simulink model "sys"  (R2024b)
settings: Solver=ode45  StartTime=0.0  StopTime=3  RelTol=1e-3
values from neighbouring files (symbols this model uses):
  N = Nm+mp*(x2+L*(theta2*cos(theta)-theta1^2*sin(theta)))    [state_equation.m]
  X = nmpc.variable(6,step)    [MPC_controller.m]    (also in MPC_controller_14D.m = nmpc.variable(12, step); mlp.m = Xy(:,1:6)')
  Y = Xy(:,7:8)'    [mlp.m]
undefined symbols (not defined in the file or in its folder): EKF, MPC_controller, Z
libraries: sm_lib x1025, nesl_utility x197, slpidlib x40, simulink x12, dspfdesign x2
(part 26 of 26)

== matlab function sys/Subsystem9/MATLAB Function2 ==
  data: q1 input; theta output; q2 input; phi input
function theta = fcn(q1, q2, phi)

alp = (q2-q1)/2;
theta = alp + q1 -phi;

== matlab function sys/MATLAB Function36 ==
  data: L input; K output
function K = LQR_K(L)

    K = LQR_K(L);

== matlab function sys/MATLAB Function37 ==
  data: State input; T output; K input
function T = Controller(State,K)

    T = Controller(State,K);

== matlab function sys/Subsystem14/MATLAB Function ==
  data: q1 input; L output; q2 input
function L = fcn(q1, q2)
l1 = 0.105;
l2 = 0.125;
alp = (q2-q1)/2;
beta = asin(l1/l2*sin(alp));
theta = pi-alp-beta;
L = l2*sin(theta)/sin(alp);
% L = l1*cos(alp) + l2*cos(beta);

== matlab function sys/Subsystem14/MATLAB Function1 ==
  data: q1 input; L output; q2 input
function L = fcn(q1, q2)
l1 = 0.105;
l2 = 0.125;
alp = (q2-q1)/2;
beta = asin(l1/l2*sin(alp));
theta = pi-alp-beta;
L = l2*sin(theta)/sin(alp);

== matlab function sys/Subsystem14/MATLAB Function2 ==
  data: q1 input; theta output; q2 input; phi input
function theta = fcn(q1, q2, phi)

alp = (q2-q1)/2;
theta = alp + q1 -phi;

== matlab function sys/Subsystem14/MATLAB Function3 ==
  data: q1 input; theta output; q2 input; phi input
function theta = fcn(q1, q2, phi)

alp = (q2-q1)/2;
theta = alp + q1 -phi;

== matlab function sys/Subsystem14/MATLAB Function4 ==
  data: we input; dtheta input; theta input; dx output; dphi input; L input; dL input
function dx = fcn(we, dtheta, theta, dphi, L, dL)
    R = 0.025;
    w = we+dtheta+dphi;
    dx = w*R+L*dtheta*cos(theta)+dL*sin(theta);

== matlab function sys/Subsystem14/MATLAB Function5 ==
  data: we input; dtheta input; dx output; theta input; dphi input; L input; dL input
function dx = fcn(we, dtheta, theta, dphi, L, dL)
    R = 0.025;
    w = we+dtheta+dphi;
    dx = w*R+L*dtheta*cos(theta)+dL*sin(theta);

== matlab function sys/双腿角度差环/MATLAB Function1 ==
  settings: updateMethod=DISCRETE
  data: u input; y output
function y = fcn(u)
persistent temp;
if isempty(temp)
    temp = 0;
end
y = 0.3*u + 0.7*temp;
temp = y;

== matlab function sys/MATLAB Function44 ==
  data: T12 output; Fl input; Tp input; l input; q1 input; q2 input
function T12= VMC(Fl, Tp,l,q1,q2)
T12  = VMC(Fl,Tp,l,0.105, 0.125,q1,q2);

== matlab function sys/Subsystem9/MATLAB Function3 ==
  data: q1 input; theta output; q2 input; phi input
function theta = fcn(q1, q2, phi)

alp = (q2-q1)/2;
theta = alp + q1 -phi;

== matlab function sys/MATLAB Function45 ==
  data: T12 output; Fl input; Tp input; l input; q1 input; q2 input
function T12= VMC(Fl, Tp,l,q1,q2)
T12  = VMC(Fl,Tp,l,0.105, 0.125,q1,q2);

== matlab function sys/MATLAB Function46 ==
  data: T12 output; Fl input; Tp input; l input; q1 input; q2 input
function T12 = VMC(Fl,Tp,l,q1,q2)
T12  = VMC(Fl,Tp,l,0.105, 0.125,q1,q2);

== matlab function sys/MATLAB Function47 ==
  data: ddz_M input; F_N output; F input; Tp input; L0 input; L0_dot input; L0_ddot input; theta input; dtheta input; ddtheta input; ddz_w output
function  [F_N, ddz_w] = SupportDetection(ddz_M,F,Tp,L0,L0_dot,L0_ddot,theta,dtheta,ddtheta)

[F_N, ddz_w] = SupportDetection(ddz_M,F,Tp,L0,L0_dot,L0_ddot,theta,dtheta,ddtheta);

== matlab function sys/MATLAB Function48 ==
  data: T12 output; Fl input; Tp input; l input; q1 input; q2 input
function T12 = VMC(Fl,Tp,l,q1,q2)
T12  = VMC(Fl,Tp,l,0.105, 0.125,q1,q2);

== matlab function sys/MATLAB Function49 ==
  data: ddz_M input; F_N output; F input; Tp input; L0 input; L0_dot input; L0_ddot input; theta input; dtheta input; ddtheta input; ddz_w output
function  [F_N, ddz_w] = SupportDetection(ddz_M,F,Tp,L0,L0_dot,L0_ddot,theta,dtheta,ddtheta)

[F_N, ddz_w] = SupportDetection(ddz_M,F,Tp,L0,L0_dot,L0_ddot,theta,dtheta,ddtheta);

== matlab function sys/Subsystem12/MATLAB Function ==
  data: q1 input; L output; q2 input
function L = fcn(q1, q2)
l1 = 0.105;
l2 = 0.125;
alp = (q2-q1)/2;
beta = asin(l1/l2*sin(alp));
theta = pi-alp-beta;
L = l2*sin(theta)/sin(alp);
% L = l1*cos(alp) + l2*cos(beta);

== matlab function sys/Subsystem12/MATLAB Function1 ==
  data: q1 input; L output; q2 input
function L = fcn(q1, q2)
l1 = 0.105;
l2 = 0.125;
alp = (q2-q1)/2;
beta = asin(l1/l2*sin(alp));
theta = pi-alp-beta;
L = l2*sin(theta)/sin(alp);

== matlab function sys/Subsystem12/MATLAB Function2 ==
  data: q1 input; theta output; q2 input; phi input
function theta = fcn(q1, q2, phi)

alp = (q2-q1)/2;
theta = alp + q1 -phi;

== matlab function sys/Subsystem9/MATLAB Function4 ==
  data: we input; dtheta input; theta input; dx output; dphi input; L input; dL input
function dx = fcn(we, dtheta, theta, dphi, L, dL)
    R = 0.025;
    w = we+dtheta+dphi;
    dx = w*R+L*dtheta*cos(theta)+dL*sin(theta);

== matlab function sys/Subsystem12/MATLAB Function3 ==
  data: q1 input; theta output; q2 input; phi input
function theta = fcn(q1, q2, phi)

alp = (q2-q1)/2;
theta = alp + q1 -phi;

== matlab function sys/Subsystem12/MATLAB Function4 ==
  data: we input; dtheta input; theta input; dx output; dphi input; L input; dL input; FN input
function dx = fcn(we, dtheta, theta, dphi, L, dL, FN)
    R = 0.025;
    w = we+dtheta+dphi;
    if FN < 0.3
        w = 0;
    end
    dx = w*R+L*dtheta*cos(theta)+dL*sin(theta);

== matlab function sys/Subsystem12/MATLAB Function5 ==
  data: we input; dtheta input; dx output; theta input; dphi input; L input; dL input; FN input
function dx = fcn(we, dtheta, theta, dphi, L, dL, FN)
    R = 0.025;
    w = we+dtheta+dphi;
    if FN < 0.3
        w = 0;
    end
    dx = w*R+L*dtheta*cos(theta)+dL*sin(theta);

== matlab function sys/双腿角度差环2/MATLAB Function1 ==
  data: u input; y output
function y = fcn(u)
persistent temp;
if isempty(temp)
    temp = 0;
end
y = 0.6*u + 0.4*temp;

== matlab function sys/Subsystem13/MATLAB Function ==
  data: q1 input; L output; q2 input
function L = fcn(q1, q2)
l1 = 0.105;
l2 = 0.125;
alp = (q2-q1)/2;
beta = asin(l1/l2*sin(alp));
theta = pi-alp-beta;
L = l2*sin(theta)/sin(alp);
% L = l1*cos(alp) + l2*cos(beta);

== matlab function sys/Subsystem13/MATLAB Function1 ==
  data: q1 input; L output; q2 input
function L = fcn(q1, q2)
l1 = 0.105;
l2 = 0.125;
alp = (q2-q1)/2;
beta = asin(l1/l2*sin(alp));
theta = pi-alp-beta;
L = l2*sin(theta)/sin(alp);

== matlab function sys/Subsystem13/MATLAB Function2 ==
  data: q1 input; theta output; q2 input; phi input
function theta = fcn(q1, q2, phi)

alp = (q2-q1)/2;
theta = alp + q1 -phi;

== matlab function sys/Subsystem13/MATLAB Function3 ==
  data: q1 input; theta output; q2 input; phi input
function theta = fcn(q1, q2, phi)

alp = (q2-q1)/2;
theta = alp + q1 -phi;

== matlab function sys/Subsystem13/MATLAB Function4 ==
  data: we input; dtheta input; theta input; dx output; dphi input; L input; dL input; FN input
function dx = fcn(we, dtheta, theta, dphi, L, dL, FN)
    R = 0.025;
    w = we+dtheta+dphi;
    if FN < 0.3
        w = 0;
    end
    dx = w*R+L*dtheta*cos(theta)+dL*sin(theta);

== matlab function sys/Subsystem13/MATLAB Function5 ==
  data: we input; dtheta input; dx output; theta input; dphi input; L input; dL input; FN input
function dx = fcn(we, dtheta, theta, dphi, L, dL, FN)
    R = 0.025;
    w = we+dtheta+dphi;
    if FN < 0.3
        w = 0;
    end
    dx = w*R+L*dtheta*cos(theta)+dL*sin(theta);

== matlab function sys/双腿角度差环3/MATLAB Function1 ==
  settings: updateMethod=DISCRETE  sampleTime=0.01
  data: u input; y output
function y = fcn(u)
persistent temp;
if isempty(temp)
    temp = 0;
end
y = 0.3*u + 0.7*temp;
temp = y;

== matlab function sys/MATLAB Function50 ==
  data: u input; y output; t input
function y = fcn(u, t)
if t > 0.3
    y = u;
else
    y = 0;
end

== matlab function sys/MATLAB Function ==
  data: T12 output; F input; T input; l input; q1 input; q2 input
function T12 = VMC(F,T,l,q1,q2)
T12  = VMC(F,T,l,0.105, 0.125,q1,q2);

== matlab function sys/MATLAB Function51 ==
  data: T1 output; Fl input; Tp input; l input; q1 input; q2 input; T2 output
function [T1,T2]= VMC(Fl, Tp,l,q1,q2)
T12  = VMC(Fl,Tp,l,0.105, 0.125,q1,q2);
T1 = T12(1);
T2 = T12(2);

== matlab function sys/双腿角度差环3/MATLAB Function2 ==
  settings: updateMethod=DISCRETE  sampleTime=0.01
  data: u input; y output
function y = fcn(u)
persistent temp;
if isempty(temp)
    temp = 0;
end
y = 0.3*u + 0.7*temp;
temp = y;

== matlab function sys/MATLAB Function52 ==
  data: T1 output; Fl input; Tp input; l input; q1 input; q2 input; T2 output
function [T1,T2]= VMC(Fl, Tp,l,q1,q2)
T12  = VMC(Fl,Tp,l,0.105, 0.125,q1,q2);
T1 = T12(1);
T2 = T12(2);

== matlab function sys/MATLAB Function53 ==
  data: ddz_M input; F_N output; F input; Tp input; L0 input; L0_dot input; L0_ddot input; theta input; dtheta input; ddtheta input; ddz_w output
function  [F_N, ddz_w] = SupportDetection(ddz_M,F,Tp,L0,L0_dot,L0_ddot,theta,dtheta,ddtheta)

[F_N, ddz_w] = SupportDetection(ddz_M,F,Tp,L0,L0_dot,L0_ddot,theta,dtheta,ddtheta);

== matlab function sys/MATLAB Function54 ==
  data: u input; y output; t input
function y = fcn(u, t)
if t > 0.3
    y = u;
else
    y = 0;
end

== matlab function sys/Subsystem15/MATLAB Function ==
  data: q1 input; L output; q2 input
function L = fcn(q1, q2)
l1 = 0.105;
l2 = 0.125;
alp = (q2-q1)/2;
beta = asin(l1/l2*sin(alp));
theta = pi-alp-beta;
L = l2*sin(theta)/sin(alp);
% L = l1*cos(alp) + l2*cos(beta);

== matlab function sys/Subsystem15/MATLAB Function1 ==
  data: q1 input; L output; q2 input
function L = fcn(q1, q2)
l1 = 0.105;
l2 = 0.125;
alp = (q2-q1)/2;
beta = asin(l1/l2*sin(alp));
theta = pi-alp-beta;
L = l2*sin(theta)/sin(alp);

== matlab function sys/Subsystem15/MATLAB Function2 ==
  data: q1 input; theta output; q2 input; phi input
function theta = fcn(q1, q2, phi)

alp = (q2-q1)/2;
theta = alp + q1 -phi;

== matlab function sys/Subsystem15/MATLAB Function3 ==
  data: q1 input; theta output; q2 input; phi input
function theta = fcn(q1, q2, phi)

alp = (q2-q1)/2;
theta = alp + q1 -phi;

== matlab function sys/Subsystem15/MATLAB Function4 ==
  data: we input; dtheta input; theta input; dx output; dphi input; L input; dL input; FN input
function dx = fcn(we, dtheta, theta, dphi, L, dL, FN)
    R = 0.025;
    w = we+dtheta-dphi;
    if FN < 0.3
        w = 0;
    end
    dx = w*R+L*dtheta*cos(theta)+dL*sin(theta);

== matlab function sys/Subsystem15/MATLAB Function5 ==
  data: we input; dtheta input; dx output; theta input; dphi input; L input; dL input; FN input
function dx = fcn(we, dtheta, theta, dphi, L, dL, FN)
    R = 0.025;
    w = we+dtheta-dphi;
    if FN < 0.3
        w = 0;
    end
    dx = w*R+L*dtheta*cos(theta)+dL*sin(theta);

== matlab function sys/MATLAB Function5 ==
  data: T12 output; F input; T input; l input; q1 input; q2 input
function T12 = VMC(F,T,l,q1,q2)
T12  = VMC(F,T,l,0.105, 0.125,q1,q2);

== matlab function sys/双腿角度差环4/MATLAB Function2 ==
  settings: updateMethod=DISCRETE  sampleTime=0.01
  data: u input; y output
function y = fcn(u)
persistent temp;
if isempty(temp)
    temp = 0;
end
y = 0.3*u + 0.7*temp;
temp = y;

== matlab function sys/MATLAB Function55 ==
  data: ddz_M input; F_N output; F input; Tp input; L0 input; L0_dot input; L0_ddot input; theta input; dtheta input; ddtheta input; ddz_w output
function  [F_N, ddz_w] = SupportDetection(ddz_M,F,Tp,L0,L0_dot,L0_ddot,theta,dtheta,ddtheta)

[F_N, ddz_w] = SupportDetection(ddz_M,F,Tp,L0,L0_dot,L0_ddot,theta,dtheta,ddtheta);

== matlab function sys/EKF_x2/MATLAB Function ==
  settings: updateMethod=DISCRETE
  data: acc_x input; x_estimate output; z2 input; F input; L input; sampletime data_store_memory; Q_EKF data_store_memory; R1_EKF data_store_memory; R2_EKF data_store_memory; n data_store_memory; x_hat data_store_memory; x_hat_minus data_store_memory; P data_store_memory
function x_estimate = EKF(acc_x, z2, F, L)

global sampletime Q_EKF R1_EKF R2_EKF n
global x_hat_minus x_hat P

%%%%%%%%%%%%%%%%%扩展卡尔曼滤波器%%%%%%%%%%%%%%%%%
z1 = zeros(2,1);
z1(2) = x_hat(4,1) + acc_x*sampletime;
z1(1) = x_hat(3,1) + z1(2)*sampletime;

% 计算先验状态估计
dx = Dynamics(x_hat, F, L);
x_hat_minus = x_hat + sampletime*dx;
% x_hat_minus = rk4_step(@(X) Dynamics(X, F, L0), x_hat, sampletime);

% 计算更新雅可比矩阵
A = Ja(x_hat(1),x_hat(2),x_hat(3),x_hat(4),x_hat(5),x_hat(6),L,F(1),F(2));
W = eye(6);
H_m1 = [0 0 1 0 0 0;
        0 0 0 1 0 0];
V1 = eye(2);
H_m2 = [0 0 1 0 0 0;
        0 0 0 1 0 0];
V2 = eye(2);

%% 计算先验估计误差协方差矩阵
P_minus = A*P*transpose(A) + transpose(W)*Q_EKF*W;

%第一次融合
%门限判断
gate_threshold = 4; %chi2inv(0.90,2)

S1 = H_m1*P_minus*H_m1.' + V1*R1_EKF*V1.';%测量预测协方差
inov1 = z1 - H_m1*x_hat_minus;%创新
d2_1   = inov1.'*inv(S1)*inov1;%马氏距离平方
if d2_1 <= gate_threshold
    %更新
    K =  P_minus*transpose(H_m1)/S1;
    x_hat = x_hat_minus + K*inov1;
    P = (eye(n)- K*H_m1)*P_minus;
else
    %跳过
    x_hat = x_hat_minus;
    P = P_minus;
end

% % 第二次融合
% S2 = H_m2*P*H_m2.' + V2*R2_EKF*V2.';
% inov2 = z2 - H_m2*x_hat;
% d2_2   = inov2.'*inv(S2)*inov2;
% if d2_2 <= gate_threshold
%     K =  P*transpose(H_m2)/S2;
%     x_hat = x_hat + K*inov2;
%     P = (eye(n)- K*H_m2)*P;
% end

x_estimate = x_hat(3:4);
end


function dX = Dynamics(X, f, L0)
    phi2_fun = @(Tp)Tp.*8.540925266903916e+2;
    theta2_fun = @(theta,theta1,L0,T,Tp)((T.*5.8e+3-Tp.*5.8e+3-L0.*sin(theta).*2.8449e+4+L0.*T.*cos(theta).*1.6e+5+L0.^2.*theta1.^2.*cos(theta).*sin(theta).*2.0e+3).*(-1.0./1.0e+2))./(L0.^2.*cos(theta).^2.*9.0+L0.^2.*sin(theta).^2.*2.9e+1);
    xb2_fun = @(theta,theta1,L0,T,Tp)(T.*cos(theta).*2.0e+2-Tp.*cos(theta).*2.0e+2+L0.^2.*theta1.^2.*sin(theta).^3.*1.0e+2+L0.*T.*cos(theta).^2.*8.0e+3+L0.*T.*sin(theta).^2.*8.0e+3-L0.*cos(theta).*sin(theta).*9.81e+2+L0.^2.*theta1.^2.*cos(theta).^2.*sin(theta).*1.0e+2)./(L0.*cos(theta).^2.*4.5e+1+L0.*sin(theta).^2.*1.45e+2)-L0.*theta1.^2.*sin(theta)-(L0.*cos(theta).*(T.*5.8e+3-Tp.*5.8e+3-L0.*sin(theta).*2.8449e+4+L0.*T.*cos(theta).*1.6e+5+L0.^2.*theta1.^2.*cos(theta).*sin(theta).*2.0e+3))./(L0.^2.*cos(theta).^2.*9.0e+2+L0.^2.*sin(theta).^2.*2.9e+3);

    theta2 = theta2_fun(X(1),X(2),L0,f(1),f(2));
    x2 = xb2_fun(X(1),X(2),L0,f(1),f(2));
    phi2 = phi2_fun(f(2));
    dX = [X(2);theta2;X(4);x2;X(6);phi2];
end

== matlab function sys/EKF1/MATLAB Function ==
  settings: updateMethod=DISCRETE
  data: acc_x input; x_estimate output; z2 input; F input; L input; sampletime data_store_memory; Q_EKF data_store_memory; R1_EKF data_store_memory; R2_EKF data_store_memory; n data_store_memory; x_hat data_store_memory; x_hat_minus data_store_memory; P data_store_memory
function x_estimate = EKF(acc_x, z2, F, L)

global sampletime Q_EKF R1_EKF R2_EKF n
global x_hat_minus x_hat P

%%%%%%%%%%%%%%%%%扩展卡尔曼滤波器%%%%%%%%%%%%%%%%%
z1 = zeros(2,1);
z1(2) = x_hat(4,1) + acc_x*sampletime;
z1(1) = x_hat(3,1) + z1(2)*sampletime;

% 计算先验状态估计
dx = Dynamics(x_hat, F, L);
x_hat_minus = x_hat + sampletime*dx;
% x_hat_minus = rk4_step(@(X) Dynamics(X, F, L0), x_hat, sampletime);

% 计算更新雅可比矩阵
A = Ja(x_hat(1),x_hat(2),x_hat(3),x_hat(4),x_hat(5),x_hat(6),L,F(1),F(2));
W = eye(6);
H_m1 = [0 0 1 0 0 0;
        0 0 0 1 0 0];
V1 = eye(2);
H_m2 = [0 0 1 0 0 0;
        0 0 0 1 0 0];
V2 = eye(2);

%% 计算先验估计误差协方差矩阵
P_minus = A*P*transpose(A) + transpose(W)*Q_EKF*W;

%第一次融合
%门限判断
gate_threshold = 4; %chi2inv(0.90,2)

S1 = H_m1*P_minus*H_m1.' + V1*R1_EKF*V1.';%测量预测协方差
inov1 = z1 - H_m1*x_hat_minus;%创新
d2_1   = inov1.'*inv(S1)*inov1;%马氏距离平方
if d2_1 <= gate_threshold
    %更新
    K =  P_minus*transpose(H_m1)/S1;
    x_hat = x_hat_minus + K*inov1;
    P = (eye(n)- K*H_m1)*P_minus;
else
    %跳过
    x_hat = x_hat_minus;
    P = P_minus;
end

% % 第二次融合
% S2 = H_m2*P*H_m2.' + V2*R2_EKF*V2.';
% inov2 = z2 - H_m2*x_hat;
% d2_2   = inov2.'*inv(S2)*inov2;
% if d2_2 <= gate_threshold
%     K =  P*transpose(H_m2)/S2;
%     x_hat = x_hat + K*inov2;
%     P = (eye(n)- K*H_m2)*P;
% end

x_estimate = x_hat(3:4);
end


function dX = Dynamics(X, f, L0)
    phi2_fun = @(Tp)Tp.*8.540925266903916e+2;
    theta2_fun = @(theta,theta1,L0,T,Tp)((T.*5.8e+3-Tp.*5.8e+3-L0.*sin(theta).*2.8449e+4+L0.*T.*cos(theta).*1.6e+5+L0.^2.*theta1.^2.*cos(theta).*sin(theta).*2.0e+3).*(-1.0./1.0e+2))./(L0.^2.*cos(theta).^2.*9.0+L0.^2.*sin(theta).^2.*2.9e+1);
    xb2_fun = @(theta,theta1,L0,T,Tp)(T.*cos(theta).*2.0e+2-Tp.*cos(theta).*2.0e+2+L0.^2.*theta1.^2.*sin(theta).^3.*1.0e+2+L0.*T.*cos(theta).^2.*8.0e+3+L0.*T.*sin(theta).^2.*8.0e+3-L0.*cos(theta).*sin(theta).*9.81e+2+L0.^2.*theta1.^2.*cos(theta).^2.*sin(theta).*1.0e+2)./(L0.*cos(theta).^2.*4.5e+1+L0.*sin(theta).^2.*1.45e+2)-L0.*theta1.^2.*sin(theta)-(L0.*cos(theta).*(T.*5.8e+3-Tp.*5.8e+3-L0.*sin(theta).*2.8449e+4+L0.*T.*cos(theta).*1.6e+5+L0.^2.*theta1.^2.*cos(theta).*sin(theta).*2.0e+3))./(L0.^2.*cos(theta).^2.*9.0e+2+L0.^2.*sin(theta).^2.*2.9e+3);

    theta2 = theta2_fun(X(1),X(2),L0,f(1),f(2));
    x2 = xb2_fun(X(1),X(2),L0,f(1),f(2));
    phi2 = phi2_fun(f(2));
    dX = [X(2);theta2;X(4);x2;X(6);phi2];
end
